simulink model "simulation_environment_thesis"  (R2024b)
settings: Solver=VariableStepAuto  StartTime=0.0  StopTime=3200  RelTol=1e-3
values from neighbouring files (symbols this model uses):
  A = [1, dt; 0, 1]    [estimate_and_control_fully_actuated_with_current.m]
  A1 = [1, 0, 0, 0, dt, 0 ... (first row only)    [estimate_and_control_fully_actuated_with_current.m]
  alpha = 10    [estimate_and_control_fully_actuated_with_current.m]
  alpha_xy_1 = 1    [estimate_and_control_fully_actuated_with_current.m]
  alpha_z_1 = 5    [estimate_and_control_fully_actuated_with_current.m]
  Az1 = [1, dt ... (first row only)    [estimate_and_control_fully_actuated_with_current.m]
  B = [dt; 0]    [estimate_and_control_fully_actuated_with_current.m]
  B1 = [dt, 0 ... (first row only)    [estimate_and_control_fully_actuated_with_current.m]
  Bz1 = [dt ... (first row only)    [estimate_and_control_fully_actuated_with_current.m]
  C1 = [1, 0, 0, 0, 0, 0 ... (first row only)    [estimate_and_control_fully_actuated_with_current.m]
  circumference_x = min_dist_from_ds*cos(theta)    [estimate_and_control_fully_actuated_with_current.m]
  circumference_y = min_dist_from_ds*sin(theta)    [estimate_and_control_fully_actuated_with_current.m]
  const_current = [-r; -s; 0; 0]    [estimate_and_control_fully_actuated_with_current.m]
  csi_psi_1 = 0.0003    [estimate_and_control_fully_actuated_with_current.m]
  csi_r_1 = 0.0003    [estimate_and_control_fully_actuated_with_current.m]
  csi_vcx_1 = 0.0003    [estimate_and_control_fully_actuated_with_current.m]
  csi_vcy_1 = 0.0003    [estimate_and_control_fully_actuated_with_current.m]
  csi_vcz_1 = 0.005    [estimate_and_control_fully_actuated_with_current.m]
  csi_x_1 = 0.003    [estimate_and_control_fully_actuated_with_current.m]
  csi_y_1 = 0.003    [estimate_and_control_fully_actuated_with_current.m]
  csi_z_1 = 0.005    [estimate_and_control_fully_actuated_with_current.m]
  Cz1 = [1, 0]    [estimate_and_control_fully_actuated_with_current.m]
  d = 1    [estimate_and_control_fully_actuated_with_current.m]
  delta_FOV = pi/7    (= 0.448798950513)    [estimate_and_control_fully_actuated_with_current.m]
  desired_speed = speed_0    [estimate_and_control_fully_actuated_with_current.m]
  dt = 1/Tsim    (= 0.005)    [estimate_and_control_fully_actuated_with_current.m]
  eta1 = deg2rad(0.5)    [estimate_and_control_fully_actuated_with_current.m]
  eta2 = deg2rad(0.5)    [estimate_and_control_fully_actuated_with_current.m]
  eta_psi_1 = 0.003    [estimate_and_control_fully_actuated_with_current.m]
  eta_x_1 = 0.03    [estimate_and_control_fully_actuated_with_current.m]
  eta_y_1 = 0.03    [estimate_and_control_fully_actuated_with_current.m]
  eta_z_1 = 0.005    [estimate_and_control_fully_actuated_with_current.m]
  G = dt*eye(2)    [estimate_and_control_fully_actuated_with_current.m]
  G1 = dt*eye(6)    [estimate_and_control_fully_actuated_with_current.m]
  Gz1 = dt*eye(2)    [estimate_and_control_fully_actuated_with_current.m]
  left_reference = L_x*[cos(NED_psi_ds); sin(NED_psi_ds); 0] + L_y_l*[-sin(NED_psi_ds); cos(NED_psi_ds); 0]    [estimate_and_control_fully_actuated_with_current.m]
  max_dist_from_ship = 60    [estimate_and_control_fully_actuated_with_current.m]
  min_dist_from_ds = 45    [estimate_and_control_fully_actuated_with_current.m]
  mu = -5    [estimate_and_control_fully_actuated_with_current.m]
  NED_psi_ds = -pi/2    (= -1.57079632679)    [estimate_and_control_fully_actuated_with_current.m]
  p_b1 = [-90; 90; 5]    [estimate_and_control_fully_actuated_with_current.m]
  position_t_0 = [0; 0; 0; 0]    [estimate_and_control_fully_actuated_with_current.m]
  psi_P = linspace(0,2*pi,360)'    [estimate_and_control_fully_actuated_with_current.m]
  Q = [csi1_kf^2, 0 ... (first row only)    [estimate_and_control_fully_actuated_with_current.m]
  Q1 = [csi_x_1_kf^2, 0, 0, 0, 0, 0 ... (first row only)    [estimate_and_control_fully_actuated_with_current.m]
  Qz1 = [csi_z_1_kf^2, 0 ... (first row only)    [estimate_and_control_fully_actuated_with_current.m]
  R = [eta1_kf^2, 0 ... (first row only)    [estimate_and_control_fully_actuated_with_current.m]
  R1 = [eta_x_1_kf^2, 0, 0 ... (first row only)    [estimate_and_control_fully_actuated_with_current.m]
  right_reference = L_x*[cos(NED_psi_ds); sin(NED_psi_ds); 0] + L_y_r*[sin(NED_psi_ds); -cos(NED_psi_ds); 0]    [estimate_and_control_fully_actuated_with_current.m]
  Rz1 = eta_z_1_kf^2    (= 1e-06)    [estimate_and_control_fully_actuated_with_current.m]
  speed_0 = [0.1, 0.1, 0, 0]'    [estimate_and_control_fully_actuated_with_current.m]
  tau_heave_sat = 30    [estimate_and_control_fully_actuated_with_current.m]
  tau_surge_sat = 10    [estimate_and_control_fully_actuated_with_current.m]
  tau_sway_sat = 30    [estimate_and_control_fully_actuated_with_current.m]
  tau_yaw_sat = 0.1    [estimate_and_control_fully_actuated_with_current.m]
  var_vm_x = 0.001    [estimate_and_control_fully_actuated_with_current.m]
  var_vm_y = 0.001    [estimate_and_control_fully_actuated_with_current.m]
  var_vm_z = 0.001    [estimate_and_control_fully_actuated_with_current.m]
undefined symbols (not defined in the file or in its folder): A2, alpha2, B2, csi1_2, csi2_2, csi3_2, csi4_2, csi5_2, csi6_2, csi7_2, csi8_2, eta1_2, eta2_2, eta3_2, eta4_2, eta5_2, eta6_2, G2, Q2, R2
libraries: simulink x60, simulink_extras x5
(part 4 of 16)

== system simulation_environment_thesis/PHASE 1.21/x,y,psi,r,vc estimate, XY PLANE from x,y,psi ==
  note: x_k_kprev
  note: x_knext_k
  note: ship frame
  note: [x
      | y]
  note: v
  note: doppler measurement noise
  note: u_k = v_m_k
  p : Inport
  inert_vel : Inport  Port=2
  psi : Inport  Port=3
  Constant : Constant  Value=C1
  Constant1 : Constant  Value=p_b1(1:2)
  Constant10 : Constant  Value=B1
  Constant12 : Constant  Value=const_current(1:2,1)  VectorParams1D=off
  Constant13 : Constant  Value=R1
  Constant14 : Constant  Value=A1
  Constant15 : Constant  Value=Q1
  Constant16 : Constant  Value=G1
  Constant9 : Constant  Value=A1  VectorParams1D=off
  Derivative : Derivative
  MATLAB Function : MATLAB Function  TreatAsAtomicUnit=on
  MATLAB Function10 : MATLAB Function  TreatAsAtomicUnit=on
  MATLAB Function6 : MATLAB Function  TreatAsAtomicUnit=on
  MATLAB Function7 : MATLAB Function  TreatAsAtomicUnit=on
  MATLAB Function8 : MATLAB Function  TreatAsAtomicUnit=on
  MATLAB Function9 : MATLAB Function  TreatAsAtomicUnit=on
  Mux : Mux  Inputs=3
  Mux1 : Mux  Inputs=2
  Mux2 : Mux  Inputs=2
  Mux3 : Mux  Inputs=2
  Random Number1 : RandomNumber  Variance=eta_x_1^2  Seed=1765152377  SampleTime=dt
  Random Number2 : RandomNumber  Variance=eta_y_1^2  Seed=1189379805  SampleTime=dt
  Random Number3 : RandomNumber  Variance=var_vm_x^2  Seed=335490584  SampleTime=dt
  Random Number4 : RandomNumber  Variance=var_vm_y^2  Seed=1124816114  SampleTime=dt
  Random Number8 : RandomNumber  Variance=eta_psi_1^2  Seed=769628192  SampleTime=dt
  Scope2 : Scope  NumInputPorts=5
  Selector : Selector  InputPortWidth=3  IndexOptions=Index vector (dialog)  Indices=[1 2]  OutputSizes=1
  Selector1 : Selector  InputPortWidth=6  IndexOptions=Index vector (dialog)  Indices=[1 2]  OutputSizes=1
  Selector2 : Selector  InputPortWidth=6  IndexOptions=Index vector (dialog)  Indices=[3 4 5 6]  OutputSizes=1
  Selector3 : Selector  InputPortWidth=6  IndexOptions=Index vector (dialog)  Indices=[3]  OutputSizes=1
  Selector4 : Selector  InputPortWidth=6  IndexOptions=Index vector (dialog)  Indices=[5 6]  OutputSizes=1
  Sum : Sum  Inputs=|++
  Sum5 : Sum  Inputs=|++
  Sum6 : Sum  Inputs=|+-
  Sum7 : Sum  Inputs=|++
  Sum8 : Sum  Inputs=|++-
  Unit Delay2 : UnitDelay  SampleTime=-1  HasFrameUpgradeWarning=on
  Unit Delay3 : UnitDelay  InitialCondition=alpha_xy_1*eye(6)  SampleTime=-1  HasFrameUpgradeWarning=on
  xy_est : Outport
  psi_est : Outport  Port=2
  v_c_xy : Outport  Port=3
  state_est : Outport  Port=4
  P_knext_knext : Outport  Port=5
  state_est_plane : Outport  Port=6
  wires:
  MATLAB Function7/1(x_k_k) -> Sum5/2
  Constant14/1 -> MATLAB Function10/3(A)
  Constant12/1 -> Sum8/3, Scope2/4
  Unit Delay2/1 -> Selector3/1, Selector4/1, MATLAB Function/1(u), Selector2/1, state_est_plane/1, Selector1/1, Scope2/5, Sum7/2, MATLAB Function6/1(x_k_kprev)
  Sum7/1 -> MATLAB Function7/2(u)
  Random Number4/1 -> Mux3/2
  MATLAB Function8/1(y) -> Sum5/1
  Random Number3/1 -> Mux3/1
  Constant15/1 -> MATLAB Function10/4(Q)
  MATLAB Function9/1(y) -> Sum7/1
  MATLAB Function10/2(P_knext_knext) -> Unit Delay3/1, P_knext_knext/1
  Sum8/1 -> MATLAB Function8/2(u)
  MATLAB Function10/1(K_knext) -> MATLAB Function9/1(K)
  Selector/1 -> Mux1/1, Scope2/1
  Constant9/1 -> MATLAB Function7/1(A)
  Constant13/1 -> MATLAB Function10/2(R)
  Sum6/1 -> MATLAB Function9/2(y_err)
  Constant10/1 -> MATLAB Function8/1(B)
  Unit Delay3/1 -> MATLAB Function10/1(P_k_k)
  Mux3/1 -> Sum8/1
  Constant16/1 -> MATLAB Function10/5(G)
  inert_vel/1 -> Sum8/2
  Sum5/1 -> Unit Delay2/1
  p/1 -> Selector/1
  Mux/1 -> Sum/2
  Sum/1 -> Sum6/1
  MATLAB Function6/1(pred) -> Sum6/2
  Constant/1 -> MATLAB Function6/2(C), MATLAB Function10/6(C)
  Selector1/1 -> xy_est/1
  psi/1 -> Derivative/1, Scope2/2, Mux1/2
  Mux1/1 -> Sum/1
  Selector3/1 -> psi_est/1
  Selector4/1 -> v_c_xy/1
  Derivative/1 -> Scope2/3
  Random Number2/1 -> Mux/2
  Random Number8/1 -> Mux/3
  Random Number1/1 -> Mux/1
  Constant1/1 -> MATLAB Function/2(ship_p_ds)
  MATLAB Function/1(y) -> Mux2/1
  Selector2/1 -> Mux2/2
  Mux2/1 -> state_est/1

== system simulation_environment_thesis/PHASE 1.21/z, v_c_z estimate from DEPTH measurement ==
  note: v
  note: u_k = v_m_k
  note: z_m
  p : Inport
  Constant : Constant  Value=p_b1(3)
  Constant10 : Constant  Value=Az1
  Constant11 : Constant  Value=Bz1
  Constant12 : Constant  Value=Cz1  VectorParams1D=off
  Constant13 : Constant  Value=const_current(3,1)
  Constant14 : Constant  Value=Rz1
  Constant15 : Constant  Value=Az1
  Constant16 : Constant  Value=Qz1
  Constant17 : Constant  Value=Gz1
  Constant9 : Constant  Value=Cz1  VectorParams1D=off
  Demux : Demux  Outputs=2
  Derivative1 : Derivative
  MATLAB Function : MATLAB Function  TreatAsAtomicUnit=on
  MATLAB Function1 : MATLAB Function  TreatAsAtomicUnit=on
  MATLAB Function6 : MATLAB Function  TreatAsAtomicUnit=on
  MATLAB Function7 : MATLAB Function  TreatAsAtomicUnit=on
  MATLAB Function8 : MATLAB Function  TreatAsAtomicUnit=on
  MATLAB Function9 : MATLAB Function  TreatAsAtomicUnit=on
  Mux1 : Mux  Inputs=2
  Random Number1 : RandomNumber  Variance=var_vm_z^2  Seed=1183831743  SampleTime=dt
  Scope2 : Scope  NumInputPorts=3
  Scope3 : Scope
  Selector : Selector  InputPortWidth=2  IndexOptions=Index vector (dialog)  Indices=[2]  OutputSizes=1
  Selector1 : Selector  InputPortWidth=3  IndexOptions=Index vector (dialog)  Indices=[3]  OutputSizes=1
  Sum5 : Sum  Inputs=|++
  Sum6 : Sum  Inputs=|+-
  Sum7 : Sum  Inputs=|++
  Sum8 : Sum  Inputs=|++-
  Sum9 : Sum  Inputs=|++
  Unit Delay2 : UnitDelay  SampleTime=-1  HasFrameUpgradeWarning=on
  Unit Delay3 : UnitDelay  InitialCondition=alpha_z_1*eye(2)  SampleTime=-1  HasFrameUpgradeWarning=on
  eta3 : RandomNumber  Variance=eta_z_1^2  Seed=196280499  SampleTime=dt
  z_est : Outport
  state_est : Outport  Port=2
  P_k_k : Outport  Port=3
  state_est_z : Outport  Port=4
  wires:
  Derivative1/1 -> Sum8/2
  Constant11/1 -> MATLAB Function7/1(B)
  Selector1/1 -> Derivative1/1, Scope2/1, Sum9/1
  Constant16/1 -> MATLAB Function9/5(Q)
  Demux/1 -> z_est/1
  MATLAB Function9/2(P_k_k) -> Unit Delay3/1, P_k_k/1
  Constant14/1 -> MATLAB Function9/3(R)
  Unit Delay2/1 -> Selector/1, MATLAB Function/1(u), state_est_z/1, Demux/1, Scope2/2, Sum7/2, MATLAB Function1/2(u)
  Constant12/1 -> MATLAB Function9/2(C)
  MATLAB Function1/1(y) -> Sum6/2
  MATLAB Function6/1(y) -> Sum5/2
  Sum7/1 -> MATLAB Function6/2(u)
  Random Number1/1 -> Sum8/1
  Sum6/1 -> MATLAB Function8/2(u)
  Constant9/1 -> MATLAB Function1/1(C)
  MATLAB Function9/1(K_k) -> Scope3/1, MATLAB Function8/1(K)
  Sum5/1 -> Unit Delay2/1
  Sum8/1 -> MATLAB Function7/2(u)
  Constant13/1 -> Sum8/3, Scope2/3
  MATLAB Function7/1(y) -> Sum5/1
  Sum9/1 -> Sum6/1
  Constant10/1 -> MATLAB Function6/1(A)
  MATLAB Function8/1(y) -> Sum7/1
  Constant15/1 -> MATLAB Function9/4(A)
  Constant17/1 -> MATLAB Function9/6(G)
  Unit Delay3/1 -> MATLAB Function9/1("P_k_k ")
  eta3/1 -> Sum9/2
  p/1 -> Selector1/1
  Constant/1 -> MATLAB Function/2(ship_p_ds)
  MATLAB Function/1(y) -> Mux1/1
  Selector/1 -> Mux1/2
  Mux1/1 -> state_est/1

== system simulation_environment_thesis/PHASE 1.22/CONTROLLER1 ==
  v_err : Inport
  speed_0 : Inport  Port=2
  yaw_err : Inport  Port=3
  z_err : Inport  Port=4
  ANTIWINDUP SURGE : Subsystem
  ANTIWINDUP SWAY : Subsystem
  ANTIWINDUP_HEAVE : Subsystem
  ANTIWINDUP_YAW : Subsystem
  Demux : Demux
  MATLAB Function3 : MATLAB Function  TreatAsAtomicUnit=on
  Mux : Mux
  tau : Outport
  wires:
  ANTIWINDUP SURGE/1(tau_u) -> Mux/1
  ANTIWINDUP_HEAVE/1(tau_w) -> Mux/3
  MATLAB Function3/1(K_i_u) -> ANTIWINDUP SURGE/2(K)
  MATLAB Function3/3(K_t_u) -> ANTIWINDUP SURGE/4(K_t_u)
  MATLAB Function3/2(K_p_u) -> ANTIWINDUP SURGE/3(K1)
  speed_0/1 -> MATLAB Function3/1(speed_0)
  Demux/1 -> ANTIWINDUP SURGE/1(in)
  ANTIWINDUP SWAY/1(tau_v) -> Mux/2
  v_err/1 -> Demux/1
  Mux/1 -> tau/1
  Demux/2 -> ANTIWINDUP SWAY/1(in)
  MATLAB Function3/4(K_i_v) -> ANTIWINDUP SWAY/2(K)
  MATLAB Function3/5(K_p_v) -> ANTIWINDUP SWAY/3(K1)
  MATLAB Function3/6(K_t_v) -> ANTIWINDUP SWAY/4(K_t_v)
  ANTIWINDUP_YAW/1(tau_psi) -> Mux/4
  MATLAB Function3/14(K_t_psi) -> ANTIWINDUP_YAW/3(K_t_psi)
  MATLAB Function3/13(K_d_psi) -> ANTIWINDUP_YAW/6(K)
  Demux/4 -> ANTIWINDUP_YAW/5(in)
  MATLAB Function3/12(K_p_psi) -> ANTIWINDUP_YAW/2(K2)
  MATLAB Function3/11(K_i_psi) -> ANTIWINDUP_YAW/1(K1)
  yaw_err/1 -> ANTIWINDUP_YAW/4(in1)
  MATLAB Function3/7(K_i_w) -> ANTIWINDUP_HEAVE/1(K1)
  MATLAB Function3/8(K_p_w) -> ANTIWINDUP_HEAVE/2(K2)
  MATLAB Function3/10(K_t_w) -> ANTIWINDUP_HEAVE/3(K_t_w)
  MATLAB Function3/9(K_d_w) -> ANTIWINDUP_HEAVE/6(K)
  z_err/1 -> ANTIWINDUP_HEAVE/4(in1)
  Demux/3 -> ANTIWINDUP_HEAVE/5(in)

== system simulation_environment_thesis/PHASE 1.22/dynamic + kinematic models1 ==
  note: body frame
  note: ship frame
  note: ship frame
  tau : Inport
  ref speed : Inport  Port=2
  yaw prev : Inport  Port=3
  Constant : Constant  Value=0
  Demux3 : Demux
  Integrator : Integrator
  Integrator1 : Integrator  InitialCondition=const_current
  Integrator12 : Integrator  InitialConditionSource=external
  Integrator7 : Integrator  InitialCondition=speed_0  UpperSaturationLimit=10^3  LowerSaturationLimit=-10^3
  MATLAB Function : MATLAB Function  TreatAsAtomicUnit=on
  MATLAB Function7 : MATLAB Function  TreatAsAtomicUnit=on
  MATLAB Function9 : MATLAB Function  TreatAsAtomicUnit=on
  Mux : Mux  Inputs=3
  Mux1 : Mux  Inputs=2
  Mux2 : Mux
  Random Number : RandomNumber  Variance=csi_x_1^2  Seed=1507494249  SampleTime=dt
  Random Number1 : RandomNumber  Variance=csi_z_1^2  Seed=1810436398  SampleTime=dt
  Random Number10 : RandomNumber  Variance=csi_r_1^2  Seed=1450844074  SampleTime=dt
  Random Number2 : RandomNumber  Variance=csi_vcz_1^2  Seed=954901216  SampleTime=dt
  Random Number5 : RandomNumber  Variance=csi_y_1^2  Seed=1518976850  SampleTime=dt
  Random Number6 : RandomNumber  Variance=csi_vcx_1^2  Seed=1659030364  SampleTime=dt
  Random Number7 : RandomNumber  Variance=csi_vcy_1^2  Seed=1354165763  SampleTime=dt
  Random Number9 : RandomNumber  Variance=csi_psi_1^2  Seed=753067611  SampleTime=dt
  Selector : Selector  InputPortWidth=4  IndexOptions=Index vector (dialog)  Indices=[1 2 3]  OutputSizes=1
  Selector1 : Selector  InputPortWidth=4  IndexOptions=Index vector (dialog)  Indices=[4]  OutputSizes=1
  Selector2 : Selector  InputPortWidth=4  IndexOptions=Index vector (dialog)  Indices=[1 2 3]  OutputSizes=1
  Sum{,1..2,5} (4 alike) : Sum  Inputs=|++
  Sum4 : Sum  Inputs=|+-
  Unit Delay2 : UnitDelay  InitialCondition=position_t_0(4)  SampleTime=-1  HasFrameUpgradeWarning=on
  error speed : Outport
  v_tot inert : Outport  Port=2
  psi : Outport  Port=3
  p : Outport  Port=4
  body speed : Outport  Port=5
  wires:
  Integrator1/1 -> Sum5/2
  MATLAB Function7/1(speed_dot) -> Selector2/1, Selector1/1
  ref speed/1 -> Sum4/1
  Unit Delay2/1 -> Sum1/1, MATLAB Function9/1(yaw)
  Integrator12/1 -> Demux3/1
  MATLAB Function9/1(eta_dot) -> Integrator12/1, Sum5/1
  Sum4/1 -> error speed/1
  Sum5/1 -> Selector/1, v_tot inert/1
  yaw prev/1 -> Unit Delay2/1
  Integrator7/1 -> MATLAB Function9/2(speed), Sum4/2, body speed/1, MATLAB Function7/1(speed)
  Demux3/4 -> MATLAB Function/1("angle ")
  tau/1 -> MATLAB Function7/2(tau)
  Integrator/1 -> p/1
  MATLAB Function/1(angle) -> psi/1
  Random Number10/1 -> Sum2/2
  Random Number7/1 -> Mux2/2
  Random Number6/1 -> Mux2/1
  Random Number9/1 -> Sum1/2
  Random Number/1 -> Mux/1
  Random Number5/1 -> Mux/2
  Sum/1 -> Integrator/1
  Mux/1 -> Sum/2
  Sum1/1 -> Integrator12/2
  Random Number1/1 -> Mux/3
  Selector1/1 -> Sum2/1
  Sum2/1 -> Mux1/2
  Selector2/1 -> Mux1/1
  Mux1/1 -> Integrator7/1
  Random Number2/1 -> Mux2/3
  Mux2/1 -> Integrator1/1
  Constant/1 -> Mux2/4
  Selector/1 -> Sum/1

== system simulation_environment_thesis/PHASE 1.22/x,y,psi,r,vc estimate, XY PLANE from x,y,psi ==
  note: x_k_kprev
  note: x_knext_k
  note: ship frame
  note: [x
      | y]
  note: v
  note: doppler measurement noise
  note: u_k = v_m_k
  p : Inport
  inert_vel : Inport  Port=2
  psi : Inport  Port=3
  Constant : Constant  Value=C1
  Constant1 : Constant  Value=p_b1(1:2)
  Constant10 : Constant  Value=B1
  Constant12 : Constant  Value=const_current(1:2,1)  VectorParams1D=off
  Constant13 : Constant  Value=R1
  Constant14 : Constant  Value=A1
  Constant15 : Constant  Value=Q1
  Constant16 : Constant  Value=G1
  Constant9 : Constant  Value=A1  VectorParams1D=off
  Derivative : Derivative
  MATLAB Function : MATLAB Function  TreatAsAtomicUnit=on
  MATLAB Function10 : MATLAB Function  TreatAsAtomicUnit=on
  MATLAB Function6 : MATLAB Function  TreatAsAtomicUnit=on
  MATLAB Function7 : MATLAB Function  TreatAsAtomicUnit=on
  MATLAB Function8 : MATLAB Function  TreatAsAtomicUnit=on
  MATLAB Function9 : MATLAB Function  TreatAsAtomicUnit=on
  Mux : Mux  Inputs=3
  Mux1 : Mux  Inputs=2
  Mux2 : Mux  Inputs=2
  Mux3 : Mux  Inputs=2
  Random Number1 : RandomNumber  Variance=eta_x_1^2  Seed=1379298017  SampleTime=dt
  Random Number2 : RandomNumber  Variance=eta_y_1^2  Seed=1494372294  SampleTime=dt
  Random Number3 : RandomNumber  Variance=var_vm_x^2  Seed=1708485084  SampleTime=dt
  Random Number4 : RandomNumber  Variance=var_vm_y^2  Seed=406918835  SampleTime=dt
  Random Number8 : RandomNumber  Variance=eta_psi_1^2  Seed=876226832  SampleTime=dt
  Scope2 : Scope  NumInputPorts=5
  Selector : Selector  InputPortWidth=3  IndexOptions=Index vector (dialog)  Indices=[1 2]  OutputSizes=1
  Selector1 : Selector  InputPortWidth=6  IndexOptions=Index vector (dialog)  Indices=[1 2]  OutputSizes=1
  Selector2 : Selector  InputPortWidth=6  IndexOptions=Index vector (dialog)  Indices=[3 4 5 6]  OutputSizes=1
  Selector3 : Selector  InputPortWidth=6  IndexOptions=Index vector (dialog)  Indices=[3]  OutputSizes=1
  Selector4 : Selector  InputPortWidth=6  IndexOptions=Index vector (dialog)  Indices=[5 6]  OutputSizes=1
  Sum : Sum  Inputs=|++
  Sum5 : Sum  Inputs=|++
  Sum6 : Sum  Inputs=|+-
  Sum7 : Sum  Inputs=|++
  Sum8 : Sum  Inputs=|++-
  Unit Delay2 : UnitDelay  SampleTime=-1  HasFrameUpgradeWarning=on
  Unit Delay3 : UnitDelay  InitialCondition=alpha_xy_1*eye(6)  SampleTime=-1  HasFrameUpgradeWarning=on
  xy_est : Outport
  psi_est : Outport  Port=2
  v_c_xy : Outport  Port=3
  state_est : Outport  Port=4
  P_knext_knext : Outport  Port=5
  state_plane : Outport  Port=6
  wires:
  MATLAB Function7/1(x_k_k) -> Sum5/2
  Constant14/1 -> MATLAB Function10/3(A)
  Constant12/1 -> Sum8/3, Scope2/4
  Unit Delay2/1 -> Selector3/1, Selector4/1, MATLAB Function/1(u), Selector2/1, state_plane/1, Selector1/1, Scope2/5, Sum7/2, MATLAB Function6/1(x_k_kprev)
  Sum7/1 -> MATLAB Function7/2(u)
  Random Number4/1 -> Mux3/2
  MATLAB Function8/1(y) -> Sum5/1
  Random Number3/1 -> Mux3/1
  Constant15/1 -> MATLAB Function10/4(Q)
  MATLAB Function9/1(y) -> Sum7/1
  MATLAB Function10/2(P_knext_knext) -> Unit Delay3/1, P_knext_knext/1
  Sum8/1 -> MATLAB Function8/2(u)
  MATLAB Function10/1(K_knext) -> MATLAB Function9/1(K)
  Selector/1 -> Mux1/1, Scope2/1
  Constant9/1 -> MATLAB Function7/1(A)
  Constant13/1 -> MATLAB Function10/2(R)
  Sum6/1 -> MATLAB Function9/2(y_err)
  Constant10/1 -> MATLAB Function8/1(B)
  Unit Delay3/1 -> MATLAB Function10/1(P_k_k)
  Mux3/1 -> Sum8/1
  Constant16/1 -> MATLAB Function10/5(G)
  inert_vel/1 -> Sum8/2
  Sum5/1 -> Unit Delay2/1
  p/1 -> Selector/1
  Mux/1 -> Sum/2
  Sum/1 -> Sum6/1
  MATLAB Function6/1(pred) -> Sum6/2
  Constant/1 -> MATLAB Function6/2(C), MATLAB Function10/6(C)
  Selector1/1 -> xy_est/1
  psi/1 -> Derivative/1, Scope2/2, Mux1/2
  Mux1/1 -> Sum/1
  Selector3/1 -> psi_est/1
  Selector4/1 -> v_c_xy/1
  Derivative/1 -> Scope2/3
  Random Number2/1 -> Mux/2
  Random Number8/1 -> Mux/3
  Random Number1/1 -> Mux/1
  Constant1/1 -> MATLAB Function/2(ship_p_ds)
  MATLAB Function/1(y) -> Mux2/1
  Selector2/1 -> Mux2/2
  Mux2/1 -> state_est/1

== system simulation_environment_thesis/PHASE 1.22/z, v_c_z estimate from DEPTH measurement ==
  note: v
  note: u_k = v_m_k
  note: z_m
  p : Inport
  Constant : Constant  Value=p_b1(3)
  Constant10 : Constant  Value=Az1
  Constant11 : Constant  Value=Bz1
  Constant12 : Constant  Value=Cz1  VectorParams1D=off
  Constant13 : Constant  Value=const_current(3,1)
  Constant14 : Constant  Value=Rz1
  Constant15 : Constant  Value=Az1
  Constant16 : Constant  Value=Qz1
  Constant17 : Constant  Value=Gz1
  Constant9 : Constant  Value=Cz1  VectorParams1D=off
  Demux : Demux  Outputs=2
  Derivative1 : Derivative
  MATLAB Function : MATLAB Function  TreatAsAtomicUnit=on
  MATLAB Function1 : MATLAB Function  TreatAsAtomicUnit=on
  MATLAB Function6 : MATLAB Function  TreatAsAtomicUnit=on
  MATLAB Function7 : MATLAB Function  TreatAsAtomicUnit=on
  MATLAB Function8 : MATLAB Function  TreatAsAtomicUnit=on
  MATLAB Function9 : MATLAB Function  TreatAsAtomicUnit=on
  Mux1 : Mux  Inputs=2
  Random Number1 : RandomNumber  Variance=var_vm_z^2  Seed=58018360  SampleTime=dt
  Scope2 : Scope  NumInputPorts=3
  Scope3 : Scope
  Selector : Selector  InputPortWidth=2  IndexOptions=Index vector (dialog)  Indices=[2]  OutputSizes=1
  Selector1 : Selector  InputPortWidth=3  IndexOptions=Index vector (dialog)  Indices=[3]  OutputSizes=1
  Sum5 : Sum  Inputs=|++
  Sum6 : Sum  Inputs=|+-
  Sum7 : Sum  Inputs=|++
  Sum8 : Sum  Inputs=|++-
  Sum9 : Sum  Inputs=|++
  Unit Delay2 : UnitDelay  SampleTime=-1  HasFrameUpgradeWarning=on
  Unit Delay3 : UnitDelay  InitialCondition=alpha_z_1*eye(2)  SampleTime=-1  HasFrameUpgradeWarning=on
  eta3 : RandomNumber  Variance=eta_z_1^2  Seed=272873203  SampleTime=dt
  z_est : Outport
  state_est : Outport  Port=2
  P_k_k : Outport  Port=3
  state_z : Outport  Port=4
  wires:
  Derivative1/1 -> Sum8/2
  Constant11/1 -> MATLAB Function7/1(B)
  Selector1/1 -> Derivative1/1, Scope2/1, Sum9/1
  Constant16/1 -> MATLAB Function9/5(Q)
  Demux/1 -> z_est/1
  MATLAB Function9/2(P_k_k) -> Unit Delay3/1, P_k_k/1
  Constant14/1 -> MATLAB Function9/3(R)
  Unit Delay2/1 -> Demux/1, Selector/1, MATLAB Function/1(u), state_z/1, Scope2/2, Sum7/2, MATLAB Function1/2(u)
  Constant12/1 -> MATLAB Function9/2(C)
  MATLAB Function1/1(y) -> Sum6/2
  MATLAB Function6/1(y) -> Sum5/2
  Sum7/1 -> MATLAB Function6/2(u)
  Random Number1/1 -> Sum8/1
  Sum6/1 -> MATLAB Function8/2(u)
  Constant9/1 -> MATLAB Function1/1(C)
  MATLAB Function9/1(K_k) -> Scope3/1, MATLAB Function8/1(K)
  Sum8/1 -> MATLAB Function7/2(u)
  Constant13/1 -> Sum8/3, Scope2/3
  MATLAB Function7/1(y) -> Sum5/1
  Sum9/1 -> Sum6/1
  Constant10/1 -> MATLAB Function6/1(A)
  MATLAB Function8/1(y) -> Sum7/1
  Constant15/1 -> MATLAB Function9/4(A)
  Constant17/1 -> MATLAB Function9/6(G)
  Unit Delay3/1 -> MATLAB Function9/1("P_k_k ")
  eta3/1 -> Sum9/2
  p/1 -> Selector1/1
  Constant/1 -> MATLAB Function/2(ship_p_ds)
  MATLAB Function/1(y) -> Mux1/1
  Selector/1 -> Mux1/2
  Mux1/1 -> state_est/1
  Sum5/1 -> Unit Delay2/1

== system simulation_environment_thesis/PHASE 1.24/CONTROLLER1 ==
  v_err : Inport
  speed_0 : Inport  Port=2
  yaw_err : Inport  Port=3
  z_err : Inport  Port=4
  ANTIWINDUP SURGE : Subsystem
  ANTIWINDUP SWAY : Subsystem
  ANTIWINDUP_HEAVE : Subsystem
  ANTIWINDUP_YAW : Subsystem
  Demux : Demux
  MATLAB Function3 : MATLAB Function  TreatAsAtomicUnit=on
  Mux : Mux
  tau : Outport
  wires:
  ANTIWINDUP SURGE/1(tau_u) -> Mux/1
  ANTIWINDUP_HEAVE/1(tau_w) -> Mux/3
  MATLAB Function3/1(K_i_u) -> ANTIWINDUP SURGE/2(K)
  MATLAB Function3/3(K_t_u) -> ANTIWINDUP SURGE/4(K_t_u)
  MATLAB Function3/2(K_p_u) -> ANTIWINDUP SURGE/3(K1)
  speed_0/1 -> MATLAB Function3/1(speed_0)
  Demux/1 -> ANTIWINDUP SURGE/1(in)
  ANTIWINDUP SWAY/1(tau_v) -> Mux/2
  v_err/1 -> Demux/1
  Mux/1 -> tau/1
  Demux/2 -> ANTIWINDUP SWAY/1(in)
  MATLAB Function3/4(K_i_v) -> ANTIWINDUP SWAY/2(K)
  MATLAB Function3/5(K_p_v) -> ANTIWINDUP SWAY/3(K1)
  MATLAB Function3/6(K_t_v) -> ANTIWINDUP SWAY/4(K_t_v)
  ANTIWINDUP_YAW/1(tau_psi) -> Mux/4
  MATLAB Function3/14(K_t_psi) -> ANTIWINDUP_YAW/3(K_t_psi)
  MATLAB Function3/13(K_d_psi) -> ANTIWINDUP_YAW/6(K)
  Demux/4 -> ANTIWINDUP_YAW/5(in)
  MATLAB Function3/12(K_p_psi) -> ANTIWINDUP_YAW/2(K2)
  MATLAB Function3/11(K_i_psi) -> ANTIWINDUP_YAW/1(K1)
  yaw_err/1 -> ANTIWINDUP_YAW/4(in1)
  MATLAB Function3/7(K_i_w) -> ANTIWINDUP_HEAVE/1(K1)
  MATLAB Function3/8(K_p_w) -> ANTIWINDUP_HEAVE/2(K2)
  MATLAB Function3/10(K_t_w) -> ANTIWINDUP_HEAVE/3(K_t_w)
  MATLAB Function3/9(K_d_w) -> ANTIWINDUP_HEAVE/6(K)
  z_err/1 -> ANTIWINDUP_HEAVE/4(in1)
  Demux/3 -> ANTIWINDUP_HEAVE/5(in)

== system simulation_environment_thesis/PHASE 1.24/dynamic + kinematic models1 ==
  note: body frame
  note: ship frame
  note: ship frame
  tau : Inport
  ref speed : Inport  Port=2
  yaw prev : Inport  Port=3
  Constant : Constant  Value=0
  Demux3 : Demux
  Integrator : Integrator
  Integrator1 : Integrator  InitialCondition=const_current
  Integrator12 : Integrator  InitialConditionSource=external
  Integrator7 : Integrator  InitialCondition=speed_0  UpperSaturationLimit=10^3  LowerSaturationLimit=-10^3
  MATLAB Function : MATLAB Function  TreatAsAtomicUnit=on
  MATLAB Function7 : MATLAB Function  TreatAsAtomicUnit=on
  MATLAB Function9 : MATLAB Function  TreatAsAtomicUnit=on
  Mux : Mux  Inputs=3
  Mux1 : Mux  Inputs=2
  Mux2 : Mux
  Random Number : RandomNumber  Variance=csi_x_1^2  Seed=1946766526  SampleTime=dt
  Random Number1 : RandomNumber  Variance=csi_z_1^2  Seed=903742064  SampleTime=dt
  Random Number10 : RandomNumber  Variance=csi_r_1^2  Seed=378842527  SampleTime=dt
  Random Number2 : RandomNumber  Variance=csi_vcz_1^2  Seed=835646138  SampleTime=dt
  Random Number5 : RandomNumber  Variance=csi_y_1^2  Seed=1866054217  SampleTime=dt
  Random Number6 : RandomNumber  Variance=csi_vcx_1^2  Seed=1436569734  SampleTime=dt
  Random Number7 : RandomNumber  Variance=csi_vcy_1^2  Seed=16911773  SampleTime=dt
  Random Number9 : RandomNumber  Variance=csi_psi_1^2  Seed=1594340260  SampleTime=dt
  Selector : Selector  InputPortWidth=4  IndexOptions=Index vector (dialog)  Indices=[1 2 3]  OutputSizes=1
  Selector1 : Selector  InputPortWidth=4  IndexOptions=Index vector (dialog)  Indices=[4]  OutputSizes=1
  Selector2 : Selector  InputPortWidth=4  IndexOptions=Index vector (dialog)  Indices=[1 2 3]  OutputSizes=1
  Sum{,1..2,5} (4 alike) : Sum  Inputs=|++
  Sum4 : Sum  Inputs=|+-
  Unit Delay2 : UnitDelay  InitialCondition=position_t_0(4)  SampleTime=-1  HasFrameUpgradeWarning=on
  error speed : Outport
  v_tot inert : Outport  Port=2
  psi : Outport  Port=3
  body speed : Outport  Port=4
  p : Outport  Port=5
  wires:
  Integrator1/1 -> Sum5/2
  MATLAB Function7/1(speed_dot) -> Selector2/1, Selector1/1
  ref speed/1 -> Sum4/1
  Unit Delay2/1 -> Sum1/1, MATLAB Function9/1(yaw)
  Integrator12/1 -> Demux3/1
  MATLAB Function9/1(eta_dot) -> Integrator12/1, Sum5/1
  Sum4/1 -> error speed/1
  Sum5/1 -> Selector/1, v_tot inert/1
  yaw prev/1 -> Unit Delay2/1
  Integrator7/1 -> MATLAB Function9/2(speed), Sum4/2, body speed/1, MATLAB Function7/1(speed)
  Demux3/4 -> MATLAB Function/1("angle ")
  tau/1 -> MATLAB Function7/2(tau)
  Integrator/1 -> p/1
  MATLAB Function/1(angle) -> psi/1
  Random Number10/1 -> Sum2/2
  Random Number7/1 -> Mux2/2
  Random Number6/1 -> Mux2/1
  Random Number9/1 -> Sum1/2
  Random Number/1 -> Mux/1
  Random Number5/1 -> Mux/2
  Sum/1 -> Integrator/1
  Mux/1 -> Sum/2
  Sum1/1 -> Integrator12/2
  Random Number1/1 -> Mux/3
  Selector1/1 -> Sum2/1
  Sum2/1 -> Mux1/2
  Selector2/1 -> Mux1/1
  Mux1/1 -> Integrator7/1
  Random Number2/1 -> Mux2/3
  Mux2/1 -> Integrator1/1
  Constant/1 -> Mux2/4
  Selector/1 -> Sum/1

== system simulation_environment_thesis/PHASE 1.24/x,y,psi,r,vc estimate, XY PLANE from x,y,psi ==
  note: x_k_kprev
  note: x_knext_k
  note: ship frame
  note: [x
      | y]
  note: v
  note: doppler measurement noise
  note: u_k = v_m_k
  p : Inport
  inert_vel : Inport  Port=2
  psi : Inport  Port=3
  Constant : Constant  Value=C1
  Constant1 : Constant  Value=p_b1(1:2)
  Constant10 : Constant  Value=B1
  Constant12 : Constant  Value=const_current(1:2,1)  VectorParams1D=off
  Constant13 : Constant  Value=R1
  Constant14 : Constant  Value=A1
  Constant15 : Constant  Value=Q1
  Constant16 : Constant  Value=G1
  Constant9 : Constant  Value=A1  VectorParams1D=off
  Derivative : Derivative
  MATLAB Function : MATLAB Function  TreatAsAtomicUnit=on
  MATLAB Function10 : MATLAB Function  TreatAsAtomicUnit=on
  MATLAB Function6 : MATLAB Function  TreatAsAtomicUnit=on
  MATLAB Function7 : MATLAB Function  TreatAsAtomicUnit=on
  MATLAB Function8 : MATLAB Function  TreatAsAtomicUnit=on
  MATLAB Function9 : MATLAB Function  TreatAsAtomicUnit=on
  Mux : Mux  Inputs=3
  Mux1 : Mux  Inputs=2
  Mux2 : Mux  Inputs=2
  Mux3 : Mux  Inputs=2
  Random Number1 : RandomNumber  Variance=eta_x_1^2  Seed=197143162  SampleTime=dt
  Random Number2 : RandomNumber  Variance=eta_y_1^2  Seed=1255637574  SampleTime=dt
  Random Number3 : RandomNumber  Variance=var_vm_x^2  Seed=913377620  SampleTime=dt
  Random Number4 : RandomNumber  Variance=var_vm_y^2  Seed=129874708  SampleTime=dt
  Random Number8 : RandomNumber  Variance=eta_psi_1^2  Seed=863250481  SampleTime=dt
  Scope2 : Scope  NumInputPorts=5
  Selector : Selector  InputPortWidth=3  IndexOptions=Index vector (dialog)  Indices=[1 2]  OutputSizes=1
  Selector1 : Selector  InputPortWidth=6  IndexOptions=Index vector (dialog)  Indices=[1 2]  OutputSizes=1
  Selector2 : Selector  InputPortWidth=6  IndexOptions=Index vector (dialog)  Indices=[3 4 5 6]  OutputSizes=1
  Selector3 : Selector  InputPortWidth=6  IndexOptions=Index vector (dialog)  Indices=[3]  OutputSizes=1
  Selector4 : Selector  InputPortWidth=6  IndexOptions=Index vector (dialog)  Indices=[5 6]  OutputSizes=1
  Sum : Sum  Inputs=|++
  Sum5 : Sum  Inputs=|++
  Sum6 : Sum  Inputs=|+-
  Sum7 : Sum  Inputs=|++
  Sum8 : Sum  Inputs=|++-
  Unit Delay2 : UnitDelay  SampleTime=-1  HasFrameUpgradeWarning=on
  Unit Delay3 : UnitDelay  InitialCondition=alpha_xy_1*eye(6)  SampleTime=-1  HasFrameUpgradeWarning=on
  xy_est : Outport
  psi_est : Outport  Port=2
  v_c_xy : Outport  Port=3
  state_est : Outport  Port=4
  P_knext_knext : Outport  Port=5
  state_est_ship_fr_plane : Outport  Port=6
  wires:
  MATLAB Function7/1(x_k_k) -> Sum5/2
  Constant14/1 -> MATLAB Function10/3(A)
  Unit Delay2/1 -> Selector3/1, Selector4/1, MATLAB Function/1(u), Selector2/1, state_est_ship_fr_plane/1, Selector1/1, Scope2/5, Sum7/2, MATLAB Function6/1(x_k_kprev)
  Sum7/1 -> MATLAB Function7/2(u)
  Random Number4/1 -> Mux3/2
  MATLAB Function8/1(y) -> Sum5/1
  Random Number3/1 -> Mux3/1
  Constant15/1 -> MATLAB Function10/4(Q)
  MATLAB Function9/1(y) -> Sum7/1
  MATLAB Function10/2(P_knext_knext) -> Unit Delay3/1, P_knext_knext/1
  Sum8/1 -> MATLAB Function8/2(u)
  MATLAB Function10/1(K_knext) -> MATLAB Function9/1(K)
  Constant9/1 -> MATLAB Function7/1(A)
  Constant13/1 -> MATLAB Function10/2(R)
  Sum6/1 -> MATLAB Function9/2(y_err)
  Constant10/1 -> MATLAB Function8/1(B)
  Unit Delay3/1 -> MATLAB Function10/1(P_k_k)
  Mux3/1 -> Sum8/1
  Constant16/1 -> MATLAB Function10/5(G)
  inert_vel/1 -> Sum8/2
  Sum5/1 -> Unit Delay2/1
  p/1 -> Selector/1
  Mux/1 -> Sum/2
  Sum/1 -> Sum6/1
  MATLAB Function6/1(pred) -> Sum6/2
  Constant/1 -> MATLAB Function6/2(C), MATLAB Function10/6(C)
  Selector1/1 -> xy_est/1
  psi/1 -> Derivative/1, Scope2/2, Mux1/2
  Mux1/1 -> Sum/1
  Random Number2/1 -> Mux/2
  Random Number8/1 -> Mux/3
  Random Number1/1 -> Mux/1
  Constant1/1 -> MATLAB Function/2(ship_p_ds)
  MATLAB Function/1(y) -> Mux2/1
  Selector2/1 -> Mux2/2
  Mux2/1 -> state_est/1
  Selector3/1 -> psi_est/1
  Selector/1 -> Mux1/1, Scope2/1
  Constant12/1 -> Sum8/3, Scope2/4
  Selector4/1 -> v_c_xy/1
  Derivative/1 -> Scope2/3

== system simulation_environment_thesis/PHASE 1.24/z, v_c_z estimate from DEPTH measurement ==
  note: v
  note: u_k = v_m_k
  note: z_m
  p : Inport
  Constant : Constant  Value=p_b1(3)
  Constant10 : Constant  Value=Az1
  Constant11 : Constant  Value=Bz1
  Constant12 : Constant  Value=Cz1  VectorParams1D=off
  Constant13 : Constant  Value=const_current(3,1)
  Constant14 : Constant  Value=Rz1
  Constant15 : Constant  Value=Az1
  Constant16 : Constant  Value=Qz1
  Constant17 : Constant  Value=Gz1
  Constant9 : Constant  Value=Cz1  VectorParams1D=off
  Demux : Demux  Outputs=2
  Derivative1 : Derivative
  MATLAB Function : MATLAB Function  TreatAsAtomicUnit=on
  MATLAB Function1 : MATLAB Function  TreatAsAtomicUnit=on
  MATLAB Function6 : MATLAB Function  TreatAsAtomicUnit=on
  MATLAB Function7 : MATLAB Function  TreatAsAtomicUnit=on
  MATLAB Function8 : MATLAB Function  TreatAsAtomicUnit=on
  MATLAB Function9 : MATLAB Function  TreatAsAtomicUnit=on
  Mux1 : Mux  Inputs=2
  Random Number1 : RandomNumber  Variance=var_vm_z^2  Seed=1868843435  SampleTime=dt
  Scope2 : Scope  NumInputPorts=3
  Scope3 : Scope
  Selector : Selector  InputPortWidth=2  IndexOptions=Index vector (dialog)  Indices=[2]  OutputSizes=1
  Selector1 : Selector  InputPortWidth=3  IndexOptions=Index vector (dialog)  Indices=[3]  OutputSizes=1
  Sum5 : Sum  Inputs=|++
  Sum6 : Sum  Inputs=|+-
  Sum7 : Sum  Inputs=|++
  Sum8 : Sum  Inputs=|++-
  Sum9 : Sum  Inputs=|++
  Unit Delay2 : UnitDelay  SampleTime=-1  HasFrameUpgradeWarning=on
  Unit Delay3 : UnitDelay  InitialCondition=alpha_z_1*eye(2)  SampleTime=-1  HasFrameUpgradeWarning=on
  eta3 : RandomNumber  Variance=eta_z_1^2  Seed=407628015  SampleTime=dt
  z_est : Outport
  state_est : Outport  Port=2
  P_k_k : Outport  Port=3
  state_est_z_ship_fr : Outport  Port=4
  wires:
  Derivative1/1 -> Sum8/2
  Constant11/1 -> MATLAB Function7/1(B)
  Selector1/1 -> Derivative1/1, Scope2/1, Sum9/1
  Constant16/1 -> MATLAB Function9/5(Q)
  Demux/1 -> z_est/1
  MATLAB Function9/2(P_k_k) -> Unit Delay3/1, P_k_k/1
  Constant14/1 -> MATLAB Function9/3(R)
  Unit Delay2/1 -> Demux/1, Scope2/2, state_est_z_ship_fr/1, Selector/1, MATLAB Function/1(u), Sum7/2, MATLAB Function1/2(u)
  Constant12/1 -> MATLAB Function9/2(C)
  MATLAB Function1/1(y) -> Sum6/2
  MATLAB Function6/1(y) -> Sum5/2
  Sum7/1 -> MATLAB Function6/2(u)
  Random Number1/1 -> Sum8/1
  Sum6/1 -> MATLAB Function8/2(u)
  Constant9/1 -> MATLAB Function1/1(C)
  MATLAB Function9/1(K_k) -> Scope3/1, MATLAB Function8/1(K)
  Sum5/1 -> Unit Delay2/1
  Sum8/1 -> MATLAB Function7/2(u)
  MATLAB Function7/1(y) -> Sum5/1
  Sum9/1 -> Sum6/1
  Constant10/1 -> MATLAB Function6/1(A)
  MATLAB Function8/1(y) -> Sum7/1
  Constant15/1 -> MATLAB Function9/4(A)
  Constant17/1 -> MATLAB Function9/6(G)
  Unit Delay3/1 -> MATLAB Function9/1("P_k_k ")
  eta3/1 -> Sum9/2
  p/1 -> Selector1/1
  Constant/1 -> MATLAB Function/2(ship_p_ds)
  MATLAB Function/1(y) -> Mux1/1
  Mux1/1 -> state_est/1
  Constant13/1 -> Sum8/3, Scope2/3
  Selector/1 -> Mux1/2

== system simulation_environment_thesis/PHASE 2  /CONTROLLER ==
  v_err : Inport
  psi_err : Inport  Port=2
  speed_0 : Inport  Port=3  PortDimensions=[4,1]
  z_err : Inport  Port=4
  ANTIWINDUP SURGE : Subsystem
  ANTIWINDUP SWAY : Subsystem
  ANTIWINDUP_HEAVE : Subsystem
  ANTIWINDUP_YAW : Subsystem
  Demux : Demux
  MATLAB Function3 : MATLAB Function  TreatAsAtomicUnit=on
  Mux : Mux
  tau : Outport
  wires:
  MATLAB Function3/12(K_i_psi) -> ANTIWINDUP_YAW/1(K1)
  ANTIWINDUP SURGE/1(tau_u) -> Mux/1
  ANTIWINDUP_HEAVE/1(tau_w) -> Mux/3
  ANTIWINDUP_YAW/1(tau_psi) -> Mux/4
  MATLAB Function3/1(K_i_u) -> ANTIWINDUP SURGE/2(K)
  MATLAB Function3/3(K_t_u) -> ANTIWINDUP SURGE/4(K_t_u)
  MATLAB Function3/2(K_p_u) -> ANTIWINDUP SURGE/3(K1)
  speed_0/1 -> MATLAB Function3/1(speed_0)
  Demux/1 -> ANTIWINDUP SURGE/1(in)
  MATLAB Function3/14(K_t_psi) -> ANTIWINDUP_YAW/3(K_t_psi)
  MATLAB Function3/11(K_p_psi) -> ANTIWINDUP_YAW/2(K2)
  ANTIWINDUP SWAY/1(tau_v) -> Mux/2
  v_err/1 -> Demux/1
  Mux/1 -> tau/1
  psi_err/1 -> ANTIWINDUP_YAW/4(in1)
  Demux/2 -> ANTIWINDUP SWAY/1(in)
  MATLAB Function3/4(K_i_v) -> ANTIWINDUP SWAY/2(K)
  MATLAB Function3/5(K_p_v) -> ANTIWINDUP SWAY/3(K1)
  MATLAB Function3/6(K_t_v) -> ANTIWINDUP SWAY/4(K_t_v)
  MATLAB Function3/13(K_d_psi) -> ANTIWINDUP_YAW/6(K)
  Demux/4 -> ANTIWINDUP_YAW/5(in)
  MATLAB Function3/7(K_i_w) -> ANTIWINDUP_HEAVE/1(K1)
  MATLAB Function3/8(K_p_w) -> ANTIWINDUP_HEAVE/2(K2)
  MATLAB Function3/9(K_d_w) -> ANTIWINDUP_HEAVE/6(K)
  MATLAB Function3/10(K_t_w) -> ANTIWINDUP_HEAVE/3(K_t_w)
  Demux/3 -> ANTIWINDUP_HEAVE/5(in)
  z_err/1 -> ANTIWINDUP_HEAVE/4(in1)

== system simulation_environment_thesis/PHASE 2  /REFERENCE GENERATOR ==
  p : Inport
  v_c : Inport  Port=2
  Constant1 : Constant  Value=min_dist_from_ds
  Constant2 : Constant  Value=NED_psi_ds + pi
  Constant3 : Constant  Value=speed_0
  MATLAB Function3 : MATLAB Function  TreatAsAtomicUnit=on
  MATLAB Function7 : MATLAB Function  TreatAsAtomicUnit=on
  Memory2 : Memory
  new origin : Constant  Value=[0; 0]  VectorParams1D=off
  speed : Outport
  yaw_dock : Outport  Port=2
  stop_flag_2 : Outport  Port=3
  wires:
  p/1 -> MATLAB Function7/3(p)
  MATLAB Function7/2(stop_flag) -> stop_flag_2/1, Memory2/1
  MATLAB Function3/1(angle) -> yaw_dock/1, MATLAB Function7/5(yaw_dock)
  Constant3/1 -> MATLAB Function7/6(speed_0)
  new origin/1 -> MATLAB Function7/4(beacon)
  Constant2/1 -> MATLAB Function3/1("angle ")
  Memory2/1 -> MATLAB Function7/1("stop_flag ")
  Constant1/1 -> MATLAB Function7/7(threshold)
  MATLAB Function7/1(speed) -> speed/1
  v_c/1 -> MATLAB Function7/2(v_c)

== system simulation_environment_thesis/PHASE 2  /dynamic + kinematic models1 ==
  note: body frame
  note: ds frame
  note: body frame
  tau : Inport
  ref speed : Inport  Port=2
  last_yaw__from_ship : Inport  Port=3
  yaw_est : Inport  Port=4
  ds_p_auv : Inport  Port=5
  Constant : Constant  Value=0
  Demux : Demux
  Integrator1 : Integrator  InitialConditionSource=external
  Integrator2 : Integrator  InitialConditionSource=external
  Integrator4 : Integrator  InitialConditionSource=external  InitialCondition=speed_0  UpperSaturationLimit=10^3  LowerSaturationLimit=-10^3
  Integrator5 : Integrator  InitialCondition=const_current
  MATLAB Function : MATLAB Function  TreatAsAtomicUnit=on
  MATLAB Function7 : MATLAB Function  TreatAsAtomicUnit=on
  MATLAB Function9 : MATLAB Function  TreatAsAtomicUnit=on
  Mux : Mux  Inputs=2
  Mux1 : Mux  Inputs=2
  Mux3 : Mux  Inputs=3
  Mux4 : Mux
  Random Number : RandomNumber  Variance=csi1_2^2  Seed=71343781  SampleTime=dt
  Random Number1 : RandomNumber  Variance=csi3_2^2  Seed=658213352  SampleTime=dt
  Random Number10 : RandomNumber  Variance=csi8_2^2  Seed=163102272  SampleTime=dt
  Random Number3 : RandomNumber  Variance=csi6_2^2  Seed=967280240  SampleTime=dt
  Random Number4 : RandomNumber  Variance=csi4_2^2  Seed=284849428  SampleTime=dt
  Random Number5 : RandomNumber  Variance=csi2_2^2  Seed=649589306  SampleTime=dt
  Random Number8 : RandomNumber  Variance=csi5_2^2  Seed=923693086  SampleTime=dt
  Random Number9 : RandomNumber  Variance=csi7_2^2  Seed=1247667931  SampleTime=dt
  Selector1 : Selector  InputPortWidth=4  IndexOptions=Index vector (dialog)  Indices=[4]  OutputSizes=1
  Selector2 : Selector  InputPortWidth=4  IndexOptions=Index vector (dialog)  Indices=[1 2 3]  OutputSizes=1
  Selector3 : Selector  InputPortWidth=4  IndexOptions=Index vector (dialog)  Indices=[1 2 3]  OutputSizes=1
  Sum{,1..2,5} (4 alike) : Sum  Inputs=|++
  Sum4 : Sum  Inputs=|+-
  error speed : Outport
  v_tot ds : Outport  Port=2
  p : Outport  Port=3
  yaw : Outport  Port=4
  body speed : Outport  Port=5
  wires:
  ref speed/1 -> Integrator4/2, Sum4/1
  MATLAB Function9/1(eta_dot) -> Sum5/1, Demux/1
  tau/1 -> MATLAB Function7/2(tau)
  Sum4/1 -> error speed/1
  Sum5/1 -> Selector3/1, v_tot ds/1
  Integrator4/1 -> MATLAB Function7/1(speed), Sum4/2, body speed/1, MATLAB Function9/2(speed)
  Integrator5/1 -> Sum5/2
  last_yaw__from_ship/1 -> Integrator1/2
  Integrator1/1 -> MATLAB Function/1("angle ")
  yaw_est/1 -> MATLAB Function9/1(yaw)
  MATLAB Function/1(angle) -> Mux/2, yaw/1
  ds_p_auv/1 -> Integrator2/2
  Random Number1/1 -> Mux3/3
  Mux3/1 -> Sum/2
  Sum/1 -> Integrator2/1
  Random Number5/1 -> Mux3/2
  Random Number/1 -> Mux3/1
  Integrator2/1 -> Mux/1
  Random Number10/1 -> Sum2/2
  Selector1/1 -> Sum2/1
  Selector2/1 -> Mux1/1
  Sum2/1 -> Mux1/2
  Mux1/1 -> Integrator4/1
  MATLAB Function7/1(speed_dot) -> Selector2/1, Selector1/1
  Random Number9/1 -> Sum1/2
  Sum1/1 -> Integrator1/1
  Demux/4 -> Sum1/1
  Random Number8/1 -> Mux4/2
  Random Number4/1 -> Mux4/1
  Mux4/1 -> Integrator5/1
  Random Number3/1 -> Mux4/3
  Selector3/1 -> Sum/1
  Mux/1 -> p/1
  Constant/1 -> Mux4/4

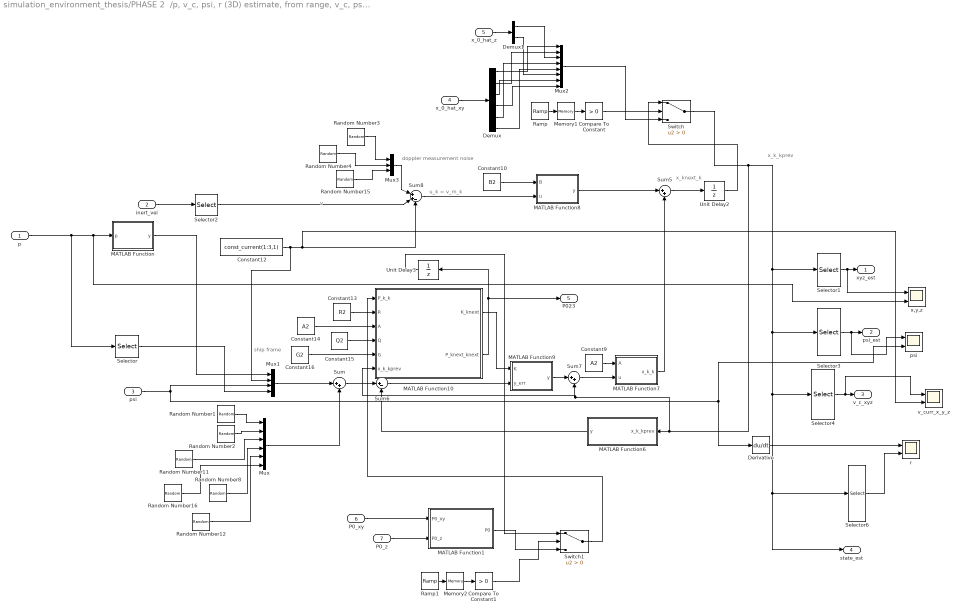
== system simulation_environment_thesis/PHASE 2  /p, v_c, psi, r (3D) estimate, from range, v_c, psi, z measurement ==
  note: x_k_kprev
  note: x_knext_k
  note: ship frame
  note: v
  note: doppler measurement noise
  note: u_k = v_m_k
  p : Inport
  inert_vel : Inport  Port=2
  psi : Inport  Port=3
  x_0_hat_xy : Inport  Port=4
  x_0_hat_z : Inport  Port=5
  P0_xy : Inport  Port=6
  P0_z : Inport  Port=7
  Compare To Constant : simulink/Logic and Bit Operations/Compare To Constant  relop=>  const=0  OutDataTypeStr=boolean
  Compare To Constant1 : simulink/Logic and Bit Operations/Compare To Constant  relop=>  const=0  OutDataTypeStr=boolean
  Constant10 : Constant  Value=B2
  Constant12 : Constant  Value=const_current(1:3,1)  VectorParams1D=off
  Constant13 : Constant  Value=R2
  Constant14 : Constant  Value=A2
  Constant15 : Constant  Value=Q2
  Constant16 : Constant  Value=G2
  Constant9 : Constant  Value=A2  VectorParams1D=off
  Demux : Demux  Outputs=6
  Demux1 : Demux  Outputs=2
  Derivative : Derivative
  MATLAB Function : MATLAB Function  TreatAsAtomicUnit=on
  MATLAB Function1 : MATLAB Function  TreatAsAtomicUnit=on
  MATLAB Function10 : MATLAB Function  TreatAsAtomicUnit=on
  MATLAB Function6 : MATLAB Function  TreatAsAtomicUnit=on
  MATLAB Function7 : MATLAB Function  TreatAsAtomicUnit=on
  MATLAB Function8 : MATLAB Function  TreatAsAtomicUnit=on
  MATLAB Function9 : MATLAB Function  TreatAsAtomicUnit=on
  Memory1 : Memory
  Memory2 : Memory
  Mux : Mux  Inputs=6
  Mux1 : Mux
  Mux2 : Mux  Inputs=8
  Mux3 : Mux  Inputs=3
  Ramp : simulink/Sources/Ramp  slope=1  start=0  InitialOutput=0
  Ramp1 : simulink/Sources/Ramp  slope=1  start=0  InitialOutput=0
  Random Number1 : RandomNumber  Variance=eta1_2^2  Seed=1819556573  SampleTime=dt
  Random Number11 : RandomNumber  Variance=eta3_2^2  Seed=1739246062  SampleTime=dt
  Random Number12 : RandomNumber  Variance=eta5_2^2  Seed=150279522  SampleTime=dt
  Random Number15 : RandomNumber  Variance=var_vm_z^2  Seed=358043529  SampleTime=dt
  Random Number16 : RandomNumber  Variance=eta6_2^2  Seed=1869970464  SampleTime=dt
  Random Number2 : RandomNumber  Variance=eta2_2^2  Seed=575434680  SampleTime=dt
  Random Number3 : RandomNumber  Variance=var_vm_x^2  Seed=1519813298  SampleTime=dt
  Random Number4 : RandomNumber  Variance=var_vm_y^2  Seed=1468984240  SampleTime=dt
  Random Number8 : RandomNumber  Variance=eta4_2^2  Seed=1543879893  SampleTime=dt
  Selector : Selector  InputPortWidth=3  IndexOptions=Index vector (dialog)  Indices=[3]  OutputSizes=1
  Selector1 : Selector  InputPortWidth=8  IndexOptions=Index vector (dialog)  Indices=[1 2 3]  OutputSizes=1
  Selector2 : Selector  InputPortWidth=4  IndexOptions=Index vector (dialog)  Indices=[1 2 3]  OutputSizes=1
  Selector3 : Selector  InputPortWidth=8  IndexOptions=Index vector (dialog)  Indices=[7]  OutputSizes=1
  Selector4 : Selector  InputPortWidth=8  IndexOptions=Index vector (dialog)  Indices=[4 5 6]  OutputSizes=1
  Selector6 : Selector  InputPortWidth=8  IndexOptions=Index vector (dialog)  Indices=[8]  OutputSizes=1
  Sum : Sum  Inputs=|++
  Sum5 : Sum  Inputs=|++
  Sum6 : Sum  Inputs=|+-
  Sum7 : Sum  Inputs=|++
  Sum8 : Sum  Inputs=|++-
  Switch : Switch  Criteria=u2 > Threshold  SaturateOnIntegerOverflow=off  InputSameDT=off
  Switch1 : Switch  Criteria=u2 > Threshold  SaturateOnIntegerOverflow=off  InputSameDT=off
  Unit Delay2 : UnitDelay  SampleTime=-1  HasFrameUpgradeWarning=on
  Unit Delay3 : UnitDelay  InitialCondition=alpha2*eye(8)  SampleTime=-1  HasFrameUpgradeWarning=on
  "psi " : Scope  NumInputPorts=2
  r : Scope  NumInputPorts=2
  v_curr_x_y_z : Scope  NumInputPorts=2
  x,y,z : Scope  NumInputPorts=2
  xyz_est : Outport
  psi_est : Outport  Port=2
  v_c_xyz : Outport  Port=3
  state_est : Outport  Port=4
  P023 : Outport  Port=5
  wires:
  MATLAB Function7/1(x_k_k) -> Sum5/2
  Constant14/1 -> MATLAB Function10/3(A)
  Sum7/1 -> MATLAB Function7/2(u)
  Random Number4/1 -> Mux3/2
  MATLAB Function8/1(y) -> Sum5/1
  Random Number3/1 -> Mux3/1
  Constant15/1 -> MATLAB Function10/4(Q)
  MATLAB Function9/1(y) -> Sum7/1
  Sum5/1 -> Unit Delay2/1
  MATLAB Function10/2(P_knext_knext) -> P023/1, Unit Delay3/1
  Sum8/1 -> MATLAB Function8/2(u)
  MATLAB Function10/1(K_knext) -> MATLAB Function9/1(K)
  Constant9/1 -> MATLAB Function7/1(A)
  Constant13/1 -> MATLAB Function10/2(R)
  Sum6/1 -> MATLAB Function9/2(y_err)
  Constant10/1 -> MATLAB Function8/1(B)
  Mux3/1 -> Sum8/1
  Constant16/1 -> MATLAB Function10/5(G)
  inert_vel/1 -> Selector2/1
  Sum/1 -> Sum6/1
  MATLAB Function6/1(y) -> Sum6/2
  Selector1/1 -> x,y,z/1, xyz_est/1
  psi/1 -> "psi "/2, Derivative/1, Mux1/3
  Mux1/1 -> Sum/1
  Selector3/1 -> "psi "/1, psi_est/1
  Selector4/1 -> v_curr_x_y_z/1, v_c_xyz/1
  Selector2/1 -> Sum8/2
  Mux/1 -> Sum/2
  Random Number2/1 -> Mux/2
  Random Number11/1 -> Mux/3
  Random Number1/1 -> Mux/1
  MATLAB Function/1(y) -> Mux1/1
  Constant12/1 -> Mux1/2, Sum8/3, v_curr_x_y_z/2
  Random Number8/1 -> Mux/4
  Random Number12/1 -> Mux/5
  Derivative/1 -> r/1
  Selector6/1 -> r/2
  Random Number15/1 -> Mux3/3
  Random Number16/1 -> Mux/6
  Selector/1 -> Mux1/4
  p/1 -> Selector/1, x,y,z/2, MATLAB Function/1(p)
  Switch/1 -> Selector3/1, Selector4/1, state_est/1, Selector6/1, Selector1/1, MATLAB Function10/6(x_k_kprev), Sum7/2, MATLAB Function6/1(x_k_kprev)
  Memory1/1 -> Compare To Constant/1
  Ramp/1 -> Memory1/1
  Compare To Constant/1 -> Switch/2
  Unit Delay2/1 -> Switch/1
  x_0_hat_z/1 -> Demux1/1
  x_0_hat_xy/1 -> Demux/1
  Demux/1 -> Mux2/1
  Demux/2 -> Mux2/2
  Demux1/1 -> Mux2/3
  Demux/5 -> Mux2/4
  Demux/6 -> Mux2/5
  Demux1/2 -> Mux2/6
  Demux/3 -> Mux2/7
  Demux/4 -> Mux2/8
  Mux2/1 -> Switch/3
  P0_xy/1 -> MATLAB Function1/1(P0_xy)
  P0_z/1 -> MATLAB Function1/2(P0_z)
  Ramp1/1 -> Memory2/1
  Memory2/1 -> Compare To Constant1/1
  Compare To Constant1/1 -> Switch1/2
  MATLAB Function1/1(P0) -> Switch1/3
  Unit Delay3/1 -> Switch1/1
  Switch1/1 -> MATLAB Function10/1(P_k_k)

== system simulation_environment_thesis/PHASE 2.21/CONTROLLER4 ==
  v_err : Inport
  psi_err : Inport  Port=2
  speed_0 : Inport  Port=3
  z_err : Inport  Port=4
  ANTIWINDUP SURGE : Subsystem
  ANTIWINDUP SWAY : Subsystem
  ANTIWINDUP_HEAVE : Subsystem
  ANTIWINDUP_YAW : Subsystem
  Demux : Demux
  MATLAB Function3 : MATLAB Function  TreatAsAtomicUnit=on
  Mux : Mux
  tau : Outport
  wires:
  MATLAB Function3/12(K_i_psi) -> ANTIWINDUP_YAW/1(K1)
  ANTIWINDUP SURGE/1(tau_u) -> Mux/1
  ANTIWINDUP_HEAVE/1(tau_w) -> Mux/3
  ANTIWINDUP_YAW/1(tau_psi) -> Mux/4
  MATLAB Function3/1(K_i_u) -> ANTIWINDUP SURGE/2(K)
  MATLAB Function3/3(K_t_u) -> ANTIWINDUP SURGE/4(K_t_u)
  MATLAB Function3/2(K_p_u) -> ANTIWINDUP SURGE/3(K1)
  speed_0/1 -> MATLAB Function3/1(speed_0)
  Demux/1 -> ANTIWINDUP SURGE/1(in)
  MATLAB Function3/14(K_t_psi) -> ANTIWINDUP_YAW/3(K_t_psi)
  MATLAB Function3/11(K_p_psi) -> ANTIWINDUP_YAW/2(K2)
  ANTIWINDUP SWAY/1(tau_v) -> Mux/2
  v_err/1 -> Demux/1
  Mux/1 -> tau/1
  psi_err/1 -> ANTIWINDUP_YAW/4(in1)
  Demux/2 -> ANTIWINDUP SWAY/1(in)
  MATLAB Function3/4(K_i_v) -> ANTIWINDUP SWAY/2(K)
  MATLAB Function3/5(K_p_v) -> ANTIWINDUP SWAY/3(K1)
  MATLAB Function3/6(K_t_v) -> ANTIWINDUP SWAY/4(K_t_v)
  MATLAB Function3/13(K_d_psi) -> ANTIWINDUP_YAW/6(K)
  Demux/4 -> ANTIWINDUP_YAW/5(in)
  MATLAB Function3/7(K_i_w) -> ANTIWINDUP_HEAVE/1(K1)
  MATLAB Function3/8(K_p_w) -> ANTIWINDUP_HEAVE/2(K2)
  MATLAB Function3/9(K_d_w) -> ANTIWINDUP_HEAVE/6(K)
  MATLAB Function3/10(K_t_w) -> ANTIWINDUP_HEAVE/3(K_t_w)
  Demux/3 -> ANTIWINDUP_HEAVE/5(in)
  z_err/1 -> ANTIWINDUP_HEAVE/4(in1)
